simulink model "Simulink_Program"  (R2023b)
settings: Solver=FixedStepAuto  StartTime=0.0  StopTime=20  FixedStep=0.001  RelTol=1e-3
PreLoadFcn:
  | % NXP_MBDT_S32K3 callback: DO NOT REMOVE
  | mbd_s32k3.common.nxp.model_callbacks.preLoadFcn();
PostLoadFcn:
  | % NXP_MBDT_S32K3 callback: DO NOT REMOVE
  | mbd_s32k3.common.nxp.model_callbacks.postLoadFcn();
InitFcn:
  | % NXP_MBDT_S32K3 callback: DO NOT REMOVE
  | mbd_s32k3.common.nxp.model_callbacks.initFcn();
undefined symbols (not defined in the file or in its folder): battery_builder_rtmsupport, DiscIntNeverNeededParam, gcbh
libraries: nesl_utility x116, pack_105s34p_structured_with_balancing_lib x105, fl_lib x16, ee_lib x7, simulink x5, BatteryBalancing x1
(part 2 of 8)

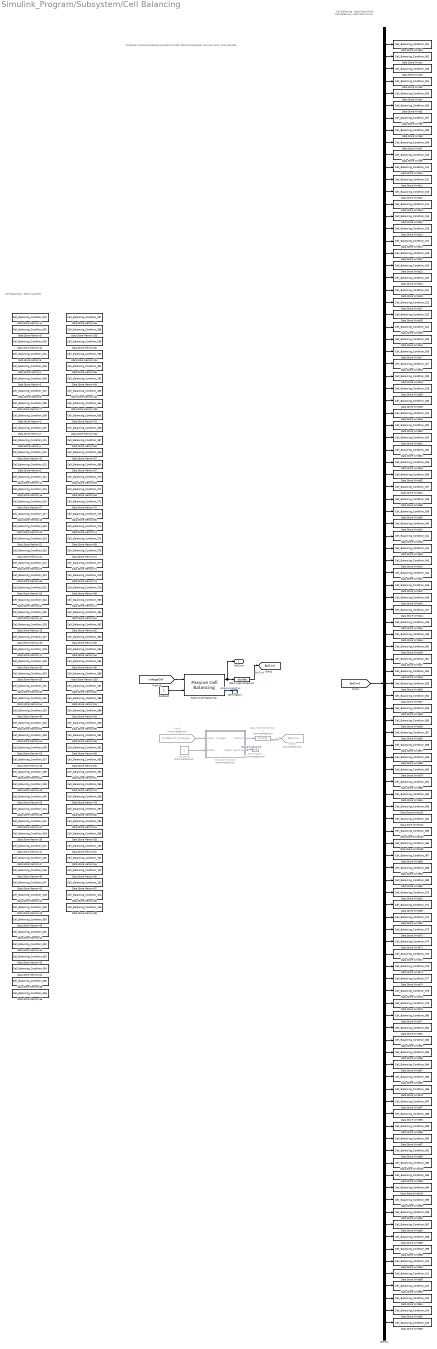
== system Simulink_Program/Subsystem/Cell Balancing ==
  note: McMaster Automotive Resource Centre (MARC)
      | Electrical Engineer: Romulo Vieira, M.Sc and MBA
  note: Cell Balancing - Store Variables
  note: Cell Balancing - Data Store Write
  note: Cell Balancing - Data Store Write
  Constant : Constant
  Constant1 : Constant  Commented=on
  Data Store Memory1 : DataStoreMemory  DataStoreName=Cell_Balancing_Condition_011  ReadBeforeWriteMsg=warning  WriteAfterWriteMsg=warning  WriteAfterReadMsg=warning  ShowAdditionalParam=off  OutDataTypeStr=double
  Data Store Memory10 : DataStoreMemory  DataStoreName=Cell_Balancing_Condition_012  ReadBeforeWriteMsg=warning  WriteAfterWriteMsg=warning  WriteAfterReadMsg=warning  ShowAdditionalParam=off  OutDataTypeStr=double
  Data Store Memory100 : DataStoreMemory  DataStoreName=Cell_Balancing_Condition_086  ReadBeforeWriteMsg=warning  WriteAfterWriteMsg=warning  WriteAfterReadMsg=warning  ShowAdditionalParam=off  OutDataTypeStr=double
  Data Store Memory101 : DataStoreMemory  DataStoreName=Cell_Balancing_Condition_063  ReadBeforeWriteMsg=warning  WriteAfterWriteMsg=warning  WriteAfterReadMsg=warning  ShowAdditionalParam=off  OutDataTypeStr=double
  Data Store Memory102 : DataStoreMemory  DataStoreName=Cell_Balancing_Condition_066  ReadBeforeWriteMsg=warning  WriteAfterWriteMsg=warning  WriteAfterReadMsg=warning  ShowAdditionalParam=off  OutDataTypeStr=double
  Data Store Memory103 : DataStoreMemory  DataStoreName=Cell_Balancing_Condition_064  ReadBeforeWriteMsg=warning  WriteAfterWriteMsg=warning  WriteAfterReadMsg=warning  ShowAdditionalParam=off  OutDataTypeStr=double
  Data Store Memory104 : DataStoreMemory  DataStoreName=Cell_Balancing_Condition_060  ReadBeforeWriteMsg=warning  WriteAfterWriteMsg=warning  WriteAfterReadMsg=warning  ShowAdditionalParam=off  OutDataTypeStr=double
  Data Store Memory105 : DataStoreMemory  DataStoreName=Cell_Balancing_Condition_058  ReadBeforeWriteMsg=warning  WriteAfterWriteMsg=warning  WriteAfterReadMsg=warning  ShowAdditionalParam=off  OutDataTypeStr=double
  Data Store Memory11 : DataStoreMemory  DataStoreName=Cell_Balancing_Condition_001  ReadBeforeWriteMsg=warning  WriteAfterWriteMsg=warning  WriteAfterReadMsg=warning  ShowAdditionalParam=off  OutDataTypeStr=double
  Data Store Memory12 : DataStoreMemory  DataStoreName=Cell_Balancing_Condition_014  ReadBeforeWriteMsg=warning  WriteAfterWriteMsg=warning  WriteAfterReadMsg=warning  ShowAdditionalParam=off  OutDataTypeStr=double
  Data Store Memory13 : DataStoreMemory  DataStoreName=Cell_Balancing_Condition_003  ReadBeforeWriteMsg=warning  WriteAfterWriteMsg=warning  WriteAfterReadMsg=warning  ShowAdditionalParam=off  OutDataTypeStr=double
  Data Store Memory14 : DataStoreMemory  DataStoreName=Cell_Balancing_Condition_025  ReadBeforeWriteMsg=warning  WriteAfterWriteMsg=warning  WriteAfterReadMsg=warning  ShowAdditionalParam=off  OutDataTypeStr=double
  Data Store Memory15 : DataStoreMemory  DataStoreName=Cell_Balancing_Condition_026  ReadBeforeWriteMsg=warning  WriteAfterWriteMsg=warning  WriteAfterReadMsg=warning  ShowAdditionalParam=off  OutDataTypeStr=double
  Data Store Memory16 : DataStoreMemory  DataStoreName=Cell_Balancing_Condition_015  ReadBeforeWriteMsg=warning  WriteAfterWriteMsg=warning  WriteAfterReadMsg=warning  ShowAdditionalParam=off  OutDataTypeStr=double
  Data Store Memory17 : DataStoreMemory  DataStoreName=Cell_Balancing_Condition_028  ReadBeforeWriteMsg=warning  WriteAfterWriteMsg=warning  WriteAfterReadMsg=warning  ShowAdditionalParam=off  OutDataTypeStr=double
  Data Store Memory18 : DataStoreMemory  DataStoreName=Cell_Balancing_Condition_017  ReadBeforeWriteMsg=warning  WriteAfterWriteMsg=warning  WriteAfterReadMsg=warning  ShowAdditionalParam=off  OutDataTypeStr=double
  Data Store Memory19 : DataStoreMemory  DataStoreName=Cell_Balancing_Condition_027  ReadBeforeWriteMsg=warning  WriteAfterWriteMsg=warning  WriteAfterReadMsg=warning  ShowAdditionalParam=off  OutDataTypeStr=double
  Data Store Memory2 : DataStoreMemory  DataStoreName=Cell_Balancing_Condition_013  ReadBeforeWriteMsg=warning  WriteAfterWriteMsg=warning  WriteAfterReadMsg=warning  ShowAdditionalParam=off  OutDataTypeStr=double
  Data Store Memory20 : DataStoreMemory  DataStoreName=Cell_Balancing_Condition_023  ReadBeforeWriteMsg=warning  WriteAfterWriteMsg=warning  WriteAfterReadMsg=warning  ShowAdditionalParam=off  OutDataTypeStr=double
  Data Store Memory21 : DataStoreMemory  DataStoreName=Cell_Balancing_Condition_019  ReadBeforeWriteMsg=warning  WriteAfterWriteMsg=warning  WriteAfterReadMsg=warning  ShowAdditionalParam=off  OutDataTypeStr=double
  Data Store Memory22 : DataStoreMemory  DataStoreName=Cell_Balancing_Condition_020  ReadBeforeWriteMsg=warning  WriteAfterWriteMsg=warning  WriteAfterReadMsg=warning  ShowAdditionalParam=off  OutDataTypeStr=double
  Data Store Memory23 : DataStoreMemory  DataStoreName=Cell_Balancing_Condition_021  ReadBeforeWriteMsg=warning  WriteAfterWriteMsg=warning  WriteAfterReadMsg=warning  ShowAdditionalParam=off  OutDataTypeStr=double
  Data Store Memory24 : DataStoreMemory  DataStoreName=Cell_Balancing_Condition_024  ReadBeforeWriteMsg=warning  WriteAfterWriteMsg=warning  WriteAfterReadMsg=warning  ShowAdditionalParam=off  OutDataTypeStr=double
  Data Store Memory25 : DataStoreMemory  DataStoreName=Cell_Balancing_Condition_022  ReadBeforeWriteMsg=warning  WriteAfterWriteMsg=warning  WriteAfterReadMsg=warning  ShowAdditionalParam=off  OutDataTypeStr=double
  Data Store Memory26 : DataStoreMemory  DataStoreName=Cell_Balancing_Condition_018  ReadBeforeWriteMsg=warning  WriteAfterWriteMsg=warning  WriteAfterReadMsg=warning  ShowAdditionalParam=off  OutDataTypeStr=double
  Data Store Memory27 : DataStoreMemory  DataStoreName=Cell_Balancing_Condition_016  ReadBeforeWriteMsg=warning  WriteAfterWriteMsg=warning  WriteAfterReadMsg=warning  ShowAdditionalParam=off  OutDataTypeStr=double
  Data Store Memory28 : DataStoreMemory  DataStoreName=Cell_Balancing_Condition_039  ReadBeforeWriteMsg=warning  WriteAfterWriteMsg=warning  WriteAfterReadMsg=warning  ShowAdditionalParam=off  OutDataTypeStr=double
  Data Store Memory29 : DataStoreMemory  DataStoreName=Cell_Balancing_Condition_040  ReadBeforeWriteMsg=warning  WriteAfterWriteMsg=warning  WriteAfterReadMsg=warning  ShowAdditionalParam=off  OutDataTypeStr=double
  Data Store Memory3 : DataStoreMemory  DataStoreName=Cell_Balancing_Condition_009  ReadBeforeWriteMsg=warning  WriteAfterWriteMsg=warning  WriteAfterReadMsg=warning  ShowAdditionalParam=off  OutDataTypeStr=double
  Data Store Memory30 : DataStoreMemory  DataStoreName=Cell_Balancing_Condition_029  ReadBeforeWriteMsg=warning  WriteAfterWriteMsg=warning  WriteAfterReadMsg=warning  ShowAdditionalParam=off  OutDataTypeStr=double
  Data Store Memory31 : DataStoreMemory  DataStoreName=Cell_Balancing_Condition_042  ReadBeforeWriteMsg=warning  WriteAfterWriteMsg=warning  WriteAfterReadMsg=warning  ShowAdditionalParam=off  OutDataTypeStr=double
  Data Store Memory32 : DataStoreMemory  DataStoreName=Cell_Balancing_Condition_031  ReadBeforeWriteMsg=warning  WriteAfterWriteMsg=warning  WriteAfterReadMsg=warning  ShowAdditionalParam=off  OutDataTypeStr=double
  Data Store Memory33 : DataStoreMemory  DataStoreName=Cell_Balancing_Condition_053  ReadBeforeWriteMsg=warning  WriteAfterWriteMsg=warning  WriteAfterReadMsg=warning  ShowAdditionalParam=off  OutDataTypeStr=double
  Data Store Memory34 : DataStoreMemory  DataStoreName=Cell_Balancing_Condition_054  ReadBeforeWriteMsg=warning  WriteAfterWriteMsg=warning  WriteAfterReadMsg=warning  ShowAdditionalParam=off  OutDataTypeStr=double
  Data Store Memory35 : DataStoreMemory  DataStoreName=Cell_Balancing_Condition_043  ReadBeforeWriteMsg=warning  WriteAfterWriteMsg=warning  WriteAfterReadMsg=warning  ShowAdditionalParam=off  OutDataTypeStr=double
  Data Store Memory36 : DataStoreMemory  DataStoreName=Cell_Balancing_Condition_056  ReadBeforeWriteMsg=warning  WriteAfterWriteMsg=warning  WriteAfterReadMsg=warning  ShowAdditionalParam=off  OutDataTypeStr=double
  Data Store Memory37 : DataStoreMemory  DataStoreName=Cell_Balancing_Condition_045  ReadBeforeWriteMsg=warning  WriteAfterWriteMsg=warning  WriteAfterReadMsg=warning  ShowAdditionalParam=off  OutDataTypeStr=double
  Data Store Memory38 : DataStoreMemory  DataStoreName=Cell_Balancing_Condition_055  ReadBeforeWriteMsg=warning  WriteAfterWriteMsg=warning  WriteAfterReadMsg=warning  ShowAdditionalParam=off  OutDataTypeStr=double
  Data Store Memory39 : DataStoreMemory  DataStoreName=Cell_Balancing_Condition_041  ReadBeforeWriteMsg=warning  WriteAfterWriteMsg=warning  WriteAfterReadMsg=warning  ShowAdditionalParam=off  OutDataTypeStr=double
  Data Store Memory4 : DataStoreMemory  DataStoreName=Cell_Balancing_Condition_005  ReadBeforeWriteMsg=warning  WriteAfterWriteMsg=warning  WriteAfterReadMsg=warning  ShowAdditionalParam=off  OutDataTypeStr=double
  Data Store Memory40 : DataStoreMemory  DataStoreName=Cell_Balancing_Condition_051  ReadBeforeWriteMsg=warning  WriteAfterWriteMsg=warning  WriteAfterReadMsg=warning  ShowAdditionalParam=off  OutDataTypeStr=double
  Data Store Memory41 : DataStoreMemory  DataStoreName=Cell_Balancing_Condition_047  ReadBeforeWriteMsg=warning  WriteAfterWriteMsg=warning  WriteAfterReadMsg=warning  ShowAdditionalParam=off  OutDataTypeStr=double
  Data Store Memory42 : DataStoreMemory  DataStoreName=Cell_Balancing_Condition_048  ReadBeforeWriteMsg=warning  WriteAfterWriteMsg=warning  WriteAfterReadMsg=warning  ShowAdditionalParam=off  OutDataTypeStr=double
  Data Store Memory43 : DataStoreMemory  DataStoreName=Cell_Balancing_Condition_049  ReadBeforeWriteMsg=warning  WriteAfterWriteMsg=warning  WriteAfterReadMsg=warning  ShowAdditionalParam=off  OutDataTypeStr=double
  Data Store Memory44 : DataStoreMemory  DataStoreName=Cell_Balancing_Condition_052  ReadBeforeWriteMsg=warning  WriteAfterWriteMsg=warning  WriteAfterReadMsg=warning  ShowAdditionalParam=off  OutDataTypeStr=double
  Data Store Memory45 : DataStoreMemory  DataStoreName=Cell_Balancing_Condition_050  ReadBeforeWriteMsg=warning  WriteAfterWriteMsg=warning  WriteAfterReadMsg=warning  ShowAdditionalParam=off  OutDataTypeStr=double
  Data Store Memory46 : DataStoreMemory  DataStoreName=Cell_Balancing_Condition_046  ReadBeforeWriteMsg=warning  WriteAfterWriteMsg=warning  WriteAfterReadMsg=warning  ShowAdditionalParam=off  OutDataTypeStr=double
  Data Store Memory47 : DataStoreMemory  DataStoreName=Cell_Balancing_Condition_044  ReadBeforeWriteMsg=warning  WriteAfterWriteMsg=warning  WriteAfterReadMsg=warning  ShowAdditionalParam=off  OutDataTypeStr=double
  Data Store Memory48 : DataStoreMemory  DataStoreName=Cell_Balancing_Condition_037  ReadBeforeWriteMsg=warning  WriteAfterWriteMsg=warning  WriteAfterReadMsg=warning  ShowAdditionalParam=off  OutDataTypeStr=double
  Data Store Memory49 : DataStoreMemory  DataStoreName=Cell_Balancing_Condition_033  ReadBeforeWriteMsg=warning  WriteAfterWriteMsg=warning  WriteAfterReadMsg=warning  ShowAdditionalParam=off  OutDataTypeStr=double
  Data Store Memory5 : DataStoreMemory  DataStoreName=Cell_Balancing_Condition_006  ReadBeforeWriteMsg=warning  WriteAfterWriteMsg=warning  WriteAfterReadMsg=warning  ShowAdditionalParam=off  OutDataTypeStr=double
  Data Store Memory50 : DataStoreMemory  DataStoreName=Cell_Balancing_Condition_034  ReadBeforeWriteMsg=warning  WriteAfterWriteMsg=warning  WriteAfterReadMsg=warning  ShowAdditionalParam=off  OutDataTypeStr=double
  Data Store Memory51 : DataStoreMemory  DataStoreName=Cell_Balancing_Condition_035  ReadBeforeWriteMsg=warning  WriteAfterWriteMsg=warning  WriteAfterReadMsg=warning  ShowAdditionalParam=off  OutDataTypeStr=double
  Data Store Memory52 : DataStoreMemory  DataStoreName=Cell_Balancing_Condition_038  ReadBeforeWriteMsg=warning  WriteAfterWriteMsg=warning  WriteAfterReadMsg=warning  ShowAdditionalParam=off  OutDataTypeStr=double
  Data Store Memory53 : DataStoreMemory  DataStoreName=Cell_Balancing_Condition_036  ReadBeforeWriteMsg=warning  WriteAfterWriteMsg=warning  WriteAfterReadMsg=warning  ShowAdditionalParam=off  OutDataTypeStr=double
  Data Store Memory54 : DataStoreMemory  DataStoreName=Cell_Balancing_Condition_032  ReadBeforeWriteMsg=warning  WriteAfterWriteMsg=warning  WriteAfterReadMsg=warning  ShowAdditionalParam=off  OutDataTypeStr=double
  Data Store Memory55 : DataStoreMemory  DataStoreName=Cell_Balancing_Condition_030  ReadBeforeWriteMsg=warning  WriteAfterWriteMsg=warning  WriteAfterReadMsg=warning  ShowAdditionalParam=off  OutDataTypeStr=double
  Data Store Memory56 : DataStoreMemory  DataStoreName=Cell_Balancing_Condition_067  ReadBeforeWriteMsg=warning  WriteAfterWriteMsg=warning  WriteAfterReadMsg=warning  ShowAdditionalParam=off  OutDataTypeStr=double
  Data Store Memory57 : DataStoreMemory  DataStoreName=Cell_Balancing_Condition_068  ReadBeforeWriteMsg=warning  WriteAfterWriteMsg=warning  WriteAfterReadMsg=warning  ShowAdditionalParam=off  OutDataTypeStr=double
  Data Store Memory58 : DataStoreMemory  DataStoreName=Cell_Balancing_Condition_057  ReadBeforeWriteMsg=warning  WriteAfterWriteMsg=warning  WriteAfterReadMsg=warning  ShowAdditionalParam=off  OutDataTypeStr=double
  Data Store Memory59 : DataStoreMemory  DataStoreName=Cell_Balancing_Condition_070  ReadBeforeWriteMsg=warning  WriteAfterWriteMsg=warning  WriteAfterReadMsg=warning  ShowAdditionalParam=off  OutDataTypeStr=double
  Data Store Memory6 : DataStoreMemory  DataStoreName=Cell_Balancing_Condition_007  ReadBeforeWriteMsg=warning  WriteAfterWriteMsg=warning  WriteAfterReadMsg=warning  ShowAdditionalParam=off  OutDataTypeStr=double
  Data Store Memory60 : DataStoreMemory  DataStoreName=Cell_Balancing_Condition_059  ReadBeforeWriteMsg=warning  WriteAfterWriteMsg=warning  WriteAfterReadMsg=warning  ShowAdditionalParam=off  OutDataTypeStr=double
  Data Store Memory61 : DataStoreMemory  DataStoreName=Cell_Balancing_Condition_081  ReadBeforeWriteMsg=warning  WriteAfterWriteMsg=warning  WriteAfterReadMsg=warning  ShowAdditionalParam=off  OutDataTypeStr=double
  Data Store Memory62 : DataStoreMemory  DataStoreName=Cell_Balancing_Condition_082  ReadBeforeWriteMsg=warning  WriteAfterWriteMsg=warning  WriteAfterReadMsg=warning  ShowAdditionalParam=off  OutDataTypeStr=double
  Data Store Memory63 : DataStoreMemory  DataStoreName=Cell_Balancing_Condition_071  ReadBeforeWriteMsg=warning  WriteAfterWriteMsg=warning  WriteAfterReadMsg=warning  ShowAdditionalParam=off  OutDataTypeStr=double
  Data Store Memory64 : DataStoreMemory  DataStoreName=Cell_Balancing_Condition_084  ReadBeforeWriteMsg=warning  WriteAfterWriteMsg=warning  WriteAfterReadMsg=warning  ShowAdditionalParam=off  OutDataTypeStr=double
  Data Store Memory65 : DataStoreMemory  DataStoreName=Cell_Balancing_Condition_073  ReadBeforeWriteMsg=warning  WriteAfterWriteMsg=warning  WriteAfterReadMsg=warning  ShowAdditionalParam=off  OutDataTypeStr=double
  Data Store Memory66 : DataStoreMemory  DataStoreName=Cell_Balancing_Condition_083  ReadBeforeWriteMsg=warning  WriteAfterWriteMsg=warning  WriteAfterReadMsg=warning  ShowAdditionalParam=off  OutDataTypeStr=double
  Data Store Memory67 : DataStoreMemory  DataStoreName=Cell_Balancing_Condition_069  ReadBeforeWriteMsg=warning  WriteAfterWriteMsg=warning  WriteAfterReadMsg=warning  ShowAdditionalParam=off  OutDataTypeStr=double
  Data Store Memory68 : DataStoreMemory  DataStoreName=Cell_Balancing_Condition_079  ReadBeforeWriteMsg=warning  WriteAfterWriteMsg=warning  WriteAfterReadMsg=warning  ShowAdditionalParam=off  OutDataTypeStr=double
  Data Store Memory69 : DataStoreMemory  DataStoreName=Cell_Balancing_Condition_075  ReadBeforeWriteMsg=warning  WriteAfterWriteMsg=warning  WriteAfterReadMsg=warning  ShowAdditionalParam=off  OutDataTypeStr=double
  Data Store Memory7 : DataStoreMemory  DataStoreName=Cell_Balancing_Condition_010  ReadBeforeWriteMsg=warning  WriteAfterWriteMsg=warning  WriteAfterReadMsg=warning  ShowAdditionalParam=off  OutDataTypeStr=double
  Data Store Memory70 : DataStoreMemory  DataStoreName=Cell_Balancing_Condition_076  ReadBeforeWriteMsg=warning  WriteAfterWriteMsg=warning  WriteAfterReadMsg=warning  ShowAdditionalParam=off  OutDataTypeStr=double
  Data Store Memory71 : DataStoreMemory  DataStoreName=Cell_Balancing_Condition_077  ReadBeforeWriteMsg=warning  WriteAfterWriteMsg=warning  WriteAfterReadMsg=warning  ShowAdditionalParam=off  OutDataTypeStr=double
  Data Store Memory72 : DataStoreMemory  DataStoreName=Cell_Balancing_Condition_080  ReadBeforeWriteMsg=warning  WriteAfterWriteMsg=warning  WriteAfterReadMsg=warning  ShowAdditionalParam=off  OutDataTypeStr=double
  Data Store Memory73 : DataStoreMemory  DataStoreName=Cell_Balancing_Condition_078  ReadBeforeWriteMsg=warning  WriteAfterWriteMsg=warning  WriteAfterReadMsg=warning  ShowAdditionalParam=off  OutDataTypeStr=double
  Data Store Memory74 : DataStoreMemory  DataStoreName=Cell_Balancing_Condition_074  ReadBeforeWriteMsg=warning  WriteAfterWriteMsg=warning  WriteAfterReadMsg=warning  ShowAdditionalParam=off  OutDataTypeStr=double
  Data Store Memory75 : DataStoreMemory  DataStoreName=Cell_Balancing_Condition_072  ReadBeforeWriteMsg=warning  WriteAfterWriteMsg=warning  WriteAfterReadMsg=warning  ShowAdditionalParam=off  OutDataTypeStr=double
  Data Store Memory76 : DataStoreMemory  DataStoreName=Cell_Balancing_Condition_095  ReadBeforeWriteMsg=warning  WriteAfterWriteMsg=warning  WriteAfterReadMsg=warning  ShowAdditionalParam=off  OutDataTypeStr=double
  Data Store Memory77 : DataStoreMemory  DataStoreName=Cell_Balancing_Condition_008  ReadBeforeWriteMsg=warning  WriteAfterWriteMsg=warning  WriteAfterReadMsg=warning  ShowAdditionalParam=off  OutDataTypeStr=double
  Data Store Memory78 : DataStoreMemory  DataStoreName=Cell_Balancing_Condition_096  ReadBeforeWriteMsg=warning  WriteAfterWriteMsg=warning  WriteAfterReadMsg=warning  ShowAdditionalParam=off  OutDataTypeStr=double
  Data Store Memory79 : DataStoreMemory  DataStoreName=Cell_Balancing_Condition_065  ReadBeforeWriteMsg=warning  WriteAfterWriteMsg=warning  WriteAfterReadMsg=warning  ShowAdditionalParam=off  OutDataTypeStr=double
  Data Store Memory8 : DataStoreMemory  DataStoreName=Cell_Balancing_Condition_004  ReadBeforeWriteMsg=warning  WriteAfterWriteMsg=warning  WriteAfterReadMsg=warning  ShowAdditionalParam=off  OutDataTypeStr=double
  Data Store Memory80 : DataStoreMemory  DataStoreName=Cell_Balancing_Condition_085  ReadBeforeWriteMsg=warning  WriteAfterWriteMsg=warning  WriteAfterReadMsg=warning  ShowAdditionalParam=off  OutDataTypeStr=double
  Data Store Memory81 : DataStoreMemory  DataStoreName=Cell_Balancing_Condition_098  ReadBeforeWriteMsg=warning  WriteAfterWriteMsg=warning  WriteAfterReadMsg=warning  ShowAdditionalParam=off  OutDataTypeStr=double
  Data Store Memory82 : DataStoreMemory  DataStoreName=Cell_Balancing_Condition_087  ReadBeforeWriteMsg=warning  WriteAfterWriteMsg=warning  WriteAfterReadMsg=warning  ShowAdditionalParam=off  OutDataTypeStr=double
  Data Store Memory83 : DataStoreMemory  DataStoreName=Cell_Balancing_Condition_099  ReadBeforeWriteMsg=warning  WriteAfterWriteMsg=warning  WriteAfterReadMsg=warning  ShowAdditionalParam=off  OutDataTypeStr=double
  Data Store Memory84 : DataStoreMemory  DataStoreName=Cell_Balancing_Condition_101  ReadBeforeWriteMsg=warning  WriteAfterWriteMsg=warning  WriteAfterReadMsg=warning  ShowAdditionalParam=off  OutDataTypeStr=double
  Data Store Memory85 : DataStoreMemory  DataStoreName=Cell_Balancing_Condition_097  ReadBeforeWriteMsg=warning  WriteAfterWriteMsg=warning  WriteAfterReadMsg=warning  ShowAdditionalParam=off  OutDataTypeStr=double
  Data Store Memory86 : DataStoreMemory  DataStoreName=Cell_Balancing_Condition_061  ReadBeforeWriteMsg=warning  WriteAfterWriteMsg=warning  WriteAfterReadMsg=warning  ShowAdditionalParam=off  OutDataTypeStr=double
  Data Store Memory87 : DataStoreMemory  DataStoreName=Cell_Balancing_Condition_103  ReadBeforeWriteMsg=warning  WriteAfterWriteMsg=warning  WriteAfterReadMsg=warning  ShowAdditionalParam=off  OutDataTypeStr=double
  Data Store Memory88 : DataStoreMemory  DataStoreName=Cell_Balancing_Condition_104  ReadBeforeWriteMsg=warning  WriteAfterWriteMsg=warning  WriteAfterReadMsg=warning  ShowAdditionalParam=off  OutDataTypeStr=double
  Data Store Memory89 : DataStoreMemory  DataStoreName=Cell_Balancing_Condition_105  ReadBeforeWriteMsg=warning  WriteAfterWriteMsg=warning  WriteAfterReadMsg=warning  ShowAdditionalParam=off  OutDataTypeStr=double
  Data Store Memory9 : DataStoreMemory  DataStoreName=Cell_Balancing_Condition_002  ReadBeforeWriteMsg=warning  WriteAfterWriteMsg=warning  WriteAfterReadMsg=warning  ShowAdditionalParam=off  OutDataTypeStr=double
  Data Store Memory90 : DataStoreMemory  DataStoreName=Cell_Balancing_Condition_102  ReadBeforeWriteMsg=warning  WriteAfterWriteMsg=warning  WriteAfterReadMsg=warning  ShowAdditionalParam=off  OutDataTypeStr=double
  Data Store Memory91 : DataStoreMemory  DataStoreName=Cell_Balancing_Condition_100  ReadBeforeWriteMsg=warning  WriteAfterWriteMsg=warning  WriteAfterReadMsg=warning  ShowAdditionalParam=off  OutDataTypeStr=double
  Data Store Memory92 : DataStoreMemory  DataStoreName=Cell_Balancing_Condition_093  ReadBeforeWriteMsg=warning  WriteAfterWriteMsg=warning  WriteAfterReadMsg=warning  ShowAdditionalParam=off  OutDataTypeStr=double
  Data Store Memory93 : DataStoreMemory  DataStoreName=Cell_Balancing_Condition_089  ReadBeforeWriteMsg=warning  WriteAfterWriteMsg=warning  WriteAfterReadMsg=warning  ShowAdditionalParam=off  OutDataTypeStr=double
  Data Store Memory94 : DataStoreMemory  DataStoreName=Cell_Balancing_Condition_062  ReadBeforeWriteMsg=warning  WriteAfterWriteMsg=warning  WriteAfterReadMsg=warning  ShowAdditionalParam=off  OutDataTypeStr=double
  Data Store Memory95 : DataStoreMemory  DataStoreName=Cell_Balancing_Condition_090  ReadBeforeWriteMsg=warning  WriteAfterWriteMsg=warning  WriteAfterReadMsg=warning  ShowAdditionalParam=off  OutDataTypeStr=double
  Data Store Memory96 : DataStoreMemory  DataStoreName=Cell_Balancing_Condition_091  ReadBeforeWriteMsg=warning  WriteAfterWriteMsg=warning  WriteAfterReadMsg=warning  ShowAdditionalParam=off  OutDataTypeStr=double
  Data Store Memory97 : DataStoreMemory  DataStoreName=Cell_Balancing_Condition_094  ReadBeforeWriteMsg=warning  WriteAfterWriteMsg=warning  WriteAfterReadMsg=warning  ShowAdditionalParam=off  OutDataTypeStr=double
  Data Store Memory98 : DataStoreMemory  DataStoreName=Cell_Balancing_Condition_092  ReadBeforeWriteMsg=warning  WriteAfterWriteMsg=warning  WriteAfterReadMsg=warning  ShowAdditionalParam=off  OutDataTypeStr=double
  Data Store Memory99 : DataStoreMemory  DataStoreName=Cell_Balancing_Condition_088  ReadBeforeWriteMsg=warning  WriteAfterWriteMsg=warning  WriteAfterReadMsg=warning  ShowAdditionalParam=off  OutDataTypeStr=double
  Data Store Write1 : DataStoreWrite  DataStoreName=Cell_Balancing_Condition_002  IndexOptions=Index vector (dialog)  Indices=1  OutputSizes=1
  Data Store Write10 : DataStoreWrite  DataStoreName=Cell_Balancing_Condition_011  IndexOptions=Index vector (dialog)  Indices=1  OutputSizes=1
  Data Store Write100 : DataStoreWrite  DataStoreName=Cell_Balancing_Condition_092  IndexOptions=Index vector (dialog)  Indices=1  OutputSizes=1
  Data Store Write101 : DataStoreWrite  DataStoreName=Cell_Balancing_Condition_094  IndexOptions=Index vector (dialog)  Indices=1  OutputSizes=1
  Data Store Write102 : DataStoreWrite  DataStoreName=Cell_Balancing_Condition_063  IndexOptions=Index vector (dialog)  Indices=1  OutputSizes=1
  Data Store Write103 : DataStoreWrite  DataStoreName=Cell_Balancing_Condition_065  IndexOptions=Index vector (dialog)  Indices=1  OutputSizes=1
  Data Store Write104 : DataStoreWrite  DataStoreName=Cell_Balancing_Condition_064  IndexOptions=Index vector (dialog)  Indices=1  OutputSizes=1
  Data Store Write105 : DataStoreWrite  DataStoreName=Cell_Balancing_Condition_066  IndexOptions=Index vector (dialog)  Indices=1  OutputSizes=1
  Data Store Write11 : DataStoreWrite  DataStoreName=Cell_Balancing_Condition_012  IndexOptions=Index vector (dialog)  Indices=1  OutputSizes=1
  Data Store Write12 : DataStoreWrite  DataStoreName=Cell_Balancing_Condition_013  IndexOptions=Index vector (dialog)  Indices=1  OutputSizes=1
  Data Store Write13 : DataStoreWrite  DataStoreName=Cell_Balancing_Condition_014  IndexOptions=Index vector (dialog)  Indices=1  OutputSizes=1
  Data Store Write14 : DataStoreWrite  DataStoreName=Cell_Balancing_Condition_016  IndexOptions=Index vector (dialog)  Indices=1  OutputSizes=1
  Data Store Write15 : DataStoreWrite  DataStoreName=Cell_Balancing_Condition_001  IndexOptions=Index vector (dialog)  Indices=1  OutputSizes=1
  Data Store Write16 : DataStoreWrite  DataStoreName=Cell_Balancing_Condition_025  IndexOptions=Index vector (dialog)  Indices=1  OutputSizes=1
  Data Store Write17 : DataStoreWrite  DataStoreName=Cell_Balancing_Condition_026  IndexOptions=Index vector (dialog)  Indices=1  OutputSizes=1
  Data Store Write18 : DataStoreWrite  DataStoreName=Cell_Balancing_Condition_027  IndexOptions=Index vector (dialog)  Indices=1  OutputSizes=1
  Data Store Write19 : DataStoreWrite  DataStoreName=Cell_Balancing_Condition_028  IndexOptions=Index vector (dialog)  Indices=1  OutputSizes=1
  Data Store Write2 : DataStoreWrite  DataStoreName=Cell_Balancing_Condition_003  IndexOptions=Index vector (dialog)  Indices=1  OutputSizes=1
  Data Store Write20 : DataStoreWrite  DataStoreName=Cell_Balancing_Condition_015  IndexOptions=Index vector (dialog)  Indices=1  OutputSizes=1
  Data Store Write21 : DataStoreWrite  DataStoreName=Cell_Balancing_Condition_017  IndexOptions=Index vector (dialog)  Indices=1  OutputSizes=1
  Data Store Write22 : DataStoreWrite  DataStoreName=Cell_Balancing_Condition_018  IndexOptions=Index vector (dialog)  Indices=1  OutputSizes=1
  Data Store Write23 : DataStoreWrite  DataStoreName=Cell_Balancing_Condition_019  IndexOptions=Index vector (dialog)  Indices=1  OutputSizes=1
  Data Store Write24 : DataStoreWrite  DataStoreName=Cell_Balancing_Condition_020  IndexOptions=Index vector (dialog)  Indices=1  OutputSizes=1
  Data Store Write25 : DataStoreWrite  DataStoreName=Cell_Balancing_Condition_021  IndexOptions=Index vector (dialog)  Indices=1  OutputSizes=1
  Data Store Write26 : DataStoreWrite  DataStoreName=Cell_Balancing_Condition_023  IndexOptions=Index vector (dialog)  Indices=1  OutputSizes=1
  Data Store Write27 : DataStoreWrite  DataStoreName=Cell_Balancing_Condition_022  IndexOptions=Index vector (dialog)  Indices=1  OutputSizes=1
  Data Store Write28 : DataStoreWrite  DataStoreName=Cell_Balancing_Condition_024  IndexOptions=Index vector (dialog)  Indices=1  OutputSizes=1
  Data Store Write29 : DataStoreWrite  DataStoreName=Cell_Balancing_Condition_030  IndexOptions=Index vector (dialog)  Indices=1  OutputSizes=1
  Data Store Write3 : DataStoreWrite  DataStoreName=Cell_Balancing_Condition_004  IndexOptions=Index vector (dialog)  Indices=1  OutputSizes=1
  Data Store Write30 : DataStoreWrite  DataStoreName=Cell_Balancing_Condition_039  IndexOptions=Index vector (dialog)  Indices=1  OutputSizes=1
  Data Store Write31 : DataStoreWrite  DataStoreName=Cell_Balancing_Condition_040  IndexOptions=Index vector (dialog)  Indices=1  OutputSizes=1
  Data Store Write32 : DataStoreWrite  DataStoreName=Cell_Balancing_Condition_041  IndexOptions=Index vector (dialog)  Indices=1  OutputSizes=1
  Data Store Write33 : DataStoreWrite  DataStoreName=Cell_Balancing_Condition_042  IndexOptions=Index vector (dialog)  Indices=1  OutputSizes=1
  Data Store Write34 : DataStoreWrite  DataStoreName=Cell_Balancing_Condition_044  IndexOptions=Index vector (dialog)  Indices=1  OutputSizes=1
  Data Store Write35 : DataStoreWrite  DataStoreName=Cell_Balancing_Condition_029  IndexOptions=Index vector (dialog)  Indices=1  OutputSizes=1
  Data Store Write36 : DataStoreWrite  DataStoreName=Cell_Balancing_Condition_053  IndexOptions=Index vector (dialog)  Indices=1  OutputSizes=1
  Data Store Write37 : DataStoreWrite  DataStoreName=Cell_Balancing_Condition_054  IndexOptions=Index vector (dialog)  Indices=1  OutputSizes=1
  Data Store Write38 : DataStoreWrite  DataStoreName=Cell_Balancing_Condition_055  IndexOptions=Index vector (dialog)  Indices=1  OutputSizes=1
  Data Store Write39 : DataStoreWrite  DataStoreName=Cell_Balancing_Condition_056  IndexOptions=Index vector (dialog)  Indices=1  OutputSizes=1
  Data Store Write4 : DataStoreWrite  DataStoreName=Cell_Balancing_Condition_005  IndexOptions=Index vector (dialog)  Indices=1  OutputSizes=1
  Data Store Write40 : DataStoreWrite  DataStoreName=Cell_Balancing_Condition_031  IndexOptions=Index vector (dialog)  Indices=1  OutputSizes=1
  Data Store Write41 : DataStoreWrite  DataStoreName=Cell_Balancing_Condition_043  IndexOptions=Index vector (dialog)  Indices=1  OutputSizes=1
  Data Store Write42 : DataStoreWrite  DataStoreName=Cell_Balancing_Condition_045  IndexOptions=Index vector (dialog)  Indices=1  OutputSizes=1
  Data Store Write43 : DataStoreWrite  DataStoreName=Cell_Balancing_Condition_046  IndexOptions=Index vector (dialog)  Indices=1  OutputSizes=1
  Data Store Write44 : DataStoreWrite  DataStoreName=Cell_Balancing_Condition_047  IndexOptions=Index vector (dialog)  Indices=1  OutputSizes=1
  Data Store Write45 : DataStoreWrite  DataStoreName=Cell_Balancing_Condition_048  IndexOptions=Index vector (dialog)  Indices=1  OutputSizes=1
  Data Store Write46 : DataStoreWrite  DataStoreName=Cell_Balancing_Condition_049  IndexOptions=Index vector (dialog)  Indices=1  OutputSizes=1
  Data Store Write47 : DataStoreWrite  DataStoreName=Cell_Balancing_Condition_051  IndexOptions=Index vector (dialog)  Indices=1  OutputSizes=1
  Data Store Write48 : DataStoreWrite  DataStoreName=Cell_Balancing_Condition_050  IndexOptions=Index vector (dialog)  Indices=1  OutputSizes=1
  Data Store Write49 : DataStoreWrite  DataStoreName=Cell_Balancing_Condition_052  IndexOptions=Index vector (dialog)  Indices=1  OutputSizes=1
  Data Store Write5 : DataStoreWrite  DataStoreName=Cell_Balancing_Condition_006  IndexOptions=Index vector (dialog)  Indices=1  OutputSizes=1
  Data Store Write50 : DataStoreWrite  DataStoreName=Cell_Balancing_Condition_032  IndexOptions=Index vector (dialog)  Indices=1  OutputSizes=1
  Data Store Write51 : DataStoreWrite  DataStoreName=Cell_Balancing_Condition_033  IndexOptions=Index vector (dialog)  Indices=1  OutputSizes=1
  Data Store Write52 : DataStoreWrite  DataStoreName=Cell_Balancing_Condition_034  IndexOptions=Index vector (dialog)  Indices=1  OutputSizes=1
  Data Store Write53 : DataStoreWrite  DataStoreName=Cell_Balancing_Condition_035  IndexOptions=Index vector (dialog)  Indices=1  OutputSizes=1
  Data Store Write54 : DataStoreWrite  DataStoreName=Cell_Balancing_Condition_037  IndexOptions=Index vector (dialog)  Indices=1  OutputSizes=1
  Data Store Write55 : DataStoreWrite  DataStoreName=Cell_Balancing_Condition_036  IndexOptions=Index vector (dialog)  Indices=1  OutputSizes=1
  Data Store Write56 : DataStoreWrite  DataStoreName=Cell_Balancing_Condition_038  IndexOptions=Index vector (dialog)  Indices=1  OutputSizes=1
  Data Store Write57 : DataStoreWrite  DataStoreName=Cell_Balancing_Condition_058  IndexOptions=Index vector (dialog)  Indices=1  OutputSizes=1
  Data Store Write58 : DataStoreWrite  DataStoreName=Cell_Balancing_Condition_067  IndexOptions=Index vector (dialog)  Indices=1  OutputSizes=1
  Data Store Write59 : DataStoreWrite  DataStoreName=Cell_Balancing_Condition_068  IndexOptions=Index vector (dialog)  Indices=1  OutputSizes=1
  Data Store Write6 : DataStoreWrite  DataStoreName=Cell_Balancing_Condition_007  IndexOptions=Index vector (dialog)  Indices=1  OutputSizes=1
  Data Store Write60 : DataStoreWrite  DataStoreName=Cell_Balancing_Condition_069  IndexOptions=Index vector (dialog)  Indices=1  OutputSizes=1
  Data Store Write61 : DataStoreWrite  DataStoreName=Cell_Balancing_Condition_070  IndexOptions=Index vector (dialog)  Indices=1  OutputSizes=1
  Data Store Write62 : DataStoreWrite  DataStoreName=Cell_Balancing_Condition_072  IndexOptions=Index vector (dialog)  Indices=1  OutputSizes=1
  Data Store Write63 : DataStoreWrite  DataStoreName=Cell_Balancing_Condition_057  IndexOptions=Index vector (dialog)  Indices=1  OutputSizes=1
  Data Store Write64 : DataStoreWrite  DataStoreName=Cell_Balancing_Condition_081  IndexOptions=Index vector (dialog)  Indices=1  OutputSizes=1
  Data Store Write65 : DataStoreWrite  DataStoreName=Cell_Balancing_Condition_082  IndexOptions=Index vector (dialog)  Indices=1  OutputSizes=1
  Data Store Write66 : DataStoreWrite  DataStoreName=Cell_Balancing_Condition_083  IndexOptions=Index vector (dialog)  Indices=1  OutputSizes=1
  Data Store Write67 : DataStoreWrite  DataStoreName=Cell_Balancing_Condition_084  IndexOptions=Index vector (dialog)  Indices=1  OutputSizes=1
  Data Store Write68 : DataStoreWrite  DataStoreName=Cell_Balancing_Condition_059  IndexOptions=Index vector (dialog)  Indices=1  OutputSizes=1
  Data Store Write69 : DataStoreWrite  DataStoreName=Cell_Balancing_Condition_071  IndexOptions=Index vector (dialog)  Indices=1  OutputSizes=1
  Data Store Write7 : DataStoreWrite  DataStoreName=Cell_Balancing_Condition_009  IndexOptions=Index vector (dialog)  Indices=1  OutputSizes=1
  Data Store Write70 : DataStoreWrite  DataStoreName=Cell_Balancing_Condition_073  IndexOptions=Index vector (dialog)  Indices=1  OutputSizes=1
  Data Store Write71 : DataStoreWrite  DataStoreName=Cell_Balancing_Condition_074  IndexOptions=Index vector (dialog)  Indices=1  OutputSizes=1
  Data Store Write72 : DataStoreWrite  DataStoreName=Cell_Balancing_Condition_075  IndexOptions=Index vector (dialog)  Indices=1  OutputSizes=1
  Data Store Write73 : DataStoreWrite  DataStoreName=Cell_Balancing_Condition_076  IndexOptions=Index vector (dialog)  Indices=1  OutputSizes=1
  Data Store Write74 : DataStoreWrite  DataStoreName=Cell_Balancing_Condition_077  IndexOptions=Index vector (dialog)  Indices=1  OutputSizes=1
  Data Store Write75 : DataStoreWrite  DataStoreName=Cell_Balancing_Condition_079  IndexOptions=Index vector (dialog)  Indices=1  OutputSizes=1
  Data Store Write76 : DataStoreWrite  DataStoreName=Cell_Balancing_Condition_078  IndexOptions=Index vector (dialog)  Indices=1  OutputSizes=1
  Data Store Write77 : DataStoreWrite  DataStoreName=Cell_Balancing_Condition_080  IndexOptions=Index vector (dialog)  Indices=1  OutputSizes=1
  Data Store Write78 : DataStoreWrite  DataStoreName=Cell_Balancing_Condition_086  IndexOptions=Index vector (dialog)  Indices=1  OutputSizes=1
  Data Store Write79 : DataStoreWrite  DataStoreName=Cell_Balancing_Condition_060  IndexOptions=Index vector (dialog)  Indices=1  OutputSizes=1
  Data Store Write8 : DataStoreWrite  DataStoreName=Cell_Balancing_Condition_008  IndexOptions=Index vector (dialog)  Indices=1  OutputSizes=1
  Data Store Write80 : DataStoreWrite  DataStoreName=Cell_Balancing_Condition_095  IndexOptions=Index vector (dialog)  Indices=1  OutputSizes=1
  Data Store Write81 : DataStoreWrite  DataStoreName=Cell_Balancing_Condition_096  IndexOptions=Index vector (dialog)  Indices=1  OutputSizes=1
  Data Store Write82 : DataStoreWrite  DataStoreName=Cell_Balancing_Condition_097  IndexOptions=Index vector (dialog)  Indices=1  OutputSizes=1
  Data Store Write83 : DataStoreWrite  DataStoreName=Cell_Balancing_Condition_098  IndexOptions=Index vector (dialog)  Indices=1  OutputSizes=1
  Data Store Write84 : DataStoreWrite  DataStoreName=Cell_Balancing_Condition_100  IndexOptions=Index vector (dialog)  Indices=1  OutputSizes=1
  Data Store Write85 : DataStoreWrite  DataStoreName=Cell_Balancing_Condition_085  IndexOptions=Index vector (dialog)  Indices=1  OutputSizes=1
  Data Store Write86 : DataStoreWrite  DataStoreName=Cell_Balancing_Condition_061  IndexOptions=Index vector (dialog)  Indices=1  OutputSizes=1
  Data Store Write87 : DataStoreWrite  DataStoreName=Cell_Balancing_Condition_087  IndexOptions=Index vector (dialog)  Indices=1  OutputSizes=1
  Data Store Write88 : DataStoreWrite  DataStoreName=Cell_Balancing_Condition_099  IndexOptions=Index vector (dialog)  Indices=1  OutputSizes=1
  Data Store Write89 : DataStoreWrite  DataStoreName=Cell_Balancing_Condition_101  IndexOptions=Index vector (dialog)  Indices=1  OutputSizes=1
  Data Store Write9 : DataStoreWrite  DataStoreName=Cell_Balancing_Condition_010  IndexOptions=Index vector (dialog)  Indices=1  OutputSizes=1
  Data Store Write90 : DataStoreWrite  DataStoreName=Cell_Balancing_Condition_102  IndexOptions=Index vector (dialog)  Indices=1  OutputSizes=1
  Data Store Write91 : DataStoreWrite  DataStoreName=Cell_Balancing_Condition_103  IndexOptions=Index vector (dialog)  Indices=1  OutputSizes=1
  Data Store Write92 : DataStoreWrite  DataStoreName=Cell_Balancing_Condition_104  IndexOptions=Index vector (dialog)  Indices=1  OutputSizes=1
  Data Store Write93 : DataStoreWrite  DataStoreName=Cell_Balancing_Condition_105  IndexOptions=Index vector (dialog)  Indices=1  OutputSizes=1
  Data Store Write94 : DataStoreWrite  DataStoreName=Cell_Balancing_Condition_062  IndexOptions=Index vector (dialog)  Indices=1  OutputSizes=1
  Data Store Write95 : DataStoreWrite  DataStoreName=Cell_Balancing_Condition_088  IndexOptions=Index vector (dialog)  Indices=1  OutputSizes=1
  Data Store Write96 : DataStoreWrite  DataStoreName=Cell_Balancing_Condition_089  IndexOptions=Index vector (dialog)  Indices=1  OutputSizes=1
  Data Store Write97 : DataStoreWrite  DataStoreName=Cell_Balancing_Condition_090  IndexOptions=Index vector (dialog)  Indices=1  OutputSizes=1
  Data Store Write98 : DataStoreWrite  DataStoreName=Cell_Balancing_Condition_091  IndexOptions=Index vector (dialog)  Indices=1  OutputSizes=1
  Data Store Write99 : DataStoreWrite  DataStoreName=Cell_Balancing_Condition_093  IndexOptions=Index vector (dialog)  Indices=1  OutputSizes=1
  Data Type Conversion : DataTypeConversion  OutDataTypeStr=double  SaturateOnIntegerOverflow=off  RndMeth=Floor
  Data Type Conversion1 : DataTypeConversion  Commented=on  OutDataTypeStr=double  SaturateOnIntegerOverflow=off  RndMeth=Floor
  Demux : Demux  Outputs=105
  From2 : From  Commented=on  GotoTag=HVBatCell_Voltages  TagVisibility=global
  Goto3 : Goto  Commented=on  GotoTag=BalCmd  TagVisibility=global
  MATLAB Function : MATLAB Function  Commented=on  TreatAsAtomicUnit=on
  Passive Cell Balancing : BatteryBalancing/Passive Cell Balancing  Threshold=0.01  HysteresisBand=0.0075  Td_on=10  Td_off=10  Ts=1  dataType=double
      settings: LibrarySourceBlock=batt_sl_lib/Cell Balancing/Passive Cell Balancing
  Terminator1 : Terminator
  Terminator2 : Terminator  Commented=on
  BalCmd : Outport
  tag scope: BalCmd=global
  wires:
  [voltageCell] -> Passive Cell Balancing/1
  Passive Cell Balancing/1 -> BalCmd/1, Data Type Conversion/1
  Constant/1 -> Passive Cell Balancing/2
  Passive Cell Balancing/2 -> Terminator1/1  "isBalancingActive"
  Data Type Conversion/1 -> [BalCmd]  "BalCmd"
  Demux/41 -> Data Store Write32/1
  Demux/11 -> Data Store Write10/1
  Demux/28 -> Data Store Write19/1
  Demux/67 -> Data Store Write58/1
  Demux/91 -> Data Store Write98/1
  Demux/22 -> Data Store Write27/1
  Demux/96 -> Data Store Write81/1
  Demux/100 -> Data Store Write84/1
  Demux/88 -> Data Store Write95/1
  Demux/95 -> Data Store Write80/1
  Demux/85 -> Data Store Write85/1
  Demux/40 -> Data Store Write31/1
  Demux/36 -> Data Store Write55/1
  Demux/3 -> Data Store Write2/1
  Demux/39 -> Data Store Write30/1
  Demux/53 -> Data Store Write36/1
  Demux/60 -> Data Store Write79/1
  Demux/18 -> Data Store Write22/1
  Demux/65 -> Data Store Write103/1
  Demux/76 -> Data Store Write73/1
  Demux/97 -> Data Store Write82/1
  [BalCmd] -> Demux/1
  Demux/70 -> Data Store Write61/1
  Demux/58 -> Data Store Write57/1
  Demux/10 -> Data Store Write9/1
  Demux/12 -> Data Store Write11/1
  Demux/101 -> Data Store Write89/1
  Demux/37 -> Data Store Write54/1
  Demux/4 -> Data Store Write3/1
  Demux/16 -> Data Store Write14/1
  Demux/19 -> Data Store Write23/1
  Demux/25 -> Data Store Write16/1
  Demux/77 -> Data Store Write74/1
  Demux/73 -> Data Store Write70/1
  Demux/75 -> Data Store Write72/1
  Demux/6 -> Data Store Write5/1
  Demux/32 -> Data Store Write50/1
  Demux/1 -> Data Store Write15/1
  Demux/66 -> Data Store Write105/1
  Demux/94 -> Data Store Write101/1
  Demux/99 -> Data Store Write88/1
  Demux/31 -> Data Store Write40/1
  Demux/45 -> Data Store Write42/1
  Demux/84 -> Data Store Write67/1
  Demux/102 -> Data Store Write90/1
  Demux/69 -> Data Store Write60/1
  Demux/71 -> Data Store Write69/1
  Demux/23 -> Data Store Write26/1
  Demux/30 -> Data Store Write29/1
  Demux/86 -> Data Store Write78/1
  Demux/54 -> Data Store Write37/1
  Demux/104 -> Data Store Write92/1
  Demux/81 -> Data Store Write64/1
  Demux/33 -> Data Store Write51/1
  Demux/21 -> Data Store Write25/1
  Demux/78 -> Data Store Write76/1
  Demux/8 -> Data Store Write8/1
  Demux/80 -> Data Store Write77/1
  Demux/5 -> Data Store Write4/1
  Demux/35 -> Data Store Write53/1
  Demux/38 -> Data Store Write56/1
  Demux/27 -> Data Store Write18/1
  Demux/29 -> Data Store Write35/1
  Demux/46 -> Data Store Write43/1
  Demux/103 -> Data Store Write91/1
  Demux/63 -> Data Store Write102/1
  Demux/20 -> Data Store Write24/1
  Demux/51 -> Data Store Write47/1
  Demux/9 -> Data Store Write7/1
  Demux/72 -> Data Store Write62/1
  Demux/50 -> Data Store Write48/1
  Demux/14 -> Data Store Write13/1
  Demux/59 -> Data Store Write68/1
  Demux/13 -> Data Store Write12/1
  Demux/34 -> Data Store Write52/1
  Demux/44 -> Data Store Write34/1
  Demux/52 -> Data Store Write49/1
  Demux/87 -> Data Store Write87/1
  Demux/7 -> Data Store Write6/1
  Demux/68 -> Data Store Write59/1
  Demux/93 -> Data Store Write99/1
  Demux/56 -> Data Store Write39/1
  Demux/17 -> Data Store Write21/1
  Demux/90 -> Data Store Write97/1
  Demux/82 -> Data Store Write65/1
  Demux/62 -> Data Store Write94/1
  Demux/74 -> Data Store Write71/1
  Demux/92 -> Data Store Write100/1
  Demux/79 -> Data Store Write75/1
  Demux/43 -> Data Store Write41/1
  Demux/57 -> Data Store Write63/1
  Demux/55 -> Data Store Write38/1
  Demux/2 -> Data Store Write1/1
  Demux/15 -> Data Store Write20/1
  Demux/61 -> Data Store Write86/1
  Demux/47 -> Data Store Write44/1
  Demux/105 -> Data Store Write93/1
  Demux/64 -> Data Store Write104/1
  Demux/48 -> Data Store Write45/1
  Demux/89 -> Data Store Write96/1
  Demux/26 -> Data Store Write17/1
  Demux/49 -> Data Store Write46/1
  Demux/98 -> Data Store Write83/1
  Demux/24 -> Data Store Write28/1
  Demux/42 -> Data Store Write33/1
  Demux/83 -> Data Store Write66/1
  Data Type Conversion1/1 -> Goto3/1  "BalCmd"
  MATLAB Function/2(isBalancingActive) -> Terminator2/1  "isBalancingActive"
  Constant1/1 -> MATLAB Function/2(Enable)
  From2/1 -> MATLAB Function/1(HVBatCell_Voltages)
  MATLAB Function/1(BalCmd) -> Data Type Conversion1/1

== system Simulink_Program/Subsystem/EVCU Received Status ==
  ChargingSysSts : Step  Time=5
  MainHVBatCntctrCmd : Step
  PlugInChargingCmd : Step  Time=0  After=0
  To Workspace : ToWorkspace  VariableName=MainHVBatCntctrCmd  SampleTime=-1
  To Workspace1 : ToWorkspace  VariableName=PlugInChargingCmd  SampleTime=-1
  To Workspace2 : ToWorkspace  VariableName=ChargingSysSts  SampleTime=-1
  " MainHVBatCntctrCmd      " : Outport
  " PlugInChargingCmd      " : Outport  Port=2
  " ChargingSysSts" : Outport  Port=3
  wires:
  MainHVBatCntctrCmd/1 -> To Workspace/1, " MainHVBatCntctrCmd      "/1
  PlugInChargingCmd/1 -> To Workspace1/1, " PlugInChargingCmd      "/1
  ChargingSysSts/1 -> To Workspace2/1, " ChargingSysSts"/1
